simulink model "l64NM_actual"  (Simulink 7.9)
settings: Solver=ode45  StartTime=0.0  StopTime=1500000  RelTol=1e-3
undefined symbols (not defined in the file): pfb_coeff_gen_calc, sfp
libraries: xbsIndex_r4 x21822, casper_library_misc x651, casper_library_delays x192, xps_library x39, xbsReplacements_r4 x16, casper_library_pfbs x16, casper_library_ffts_twiddle x8, simulink x1
(part 1 of 315)
settings Model: Creator=jkocz  LastModifiedBy=jkocz
settings DebuggingCC: FcnCallInpInsideContextMsg=Use local settings  StrictBusMsg=Warning
settings GRTTargetCC: CombineOutputUpdateFcns=off  GRTInterface=on

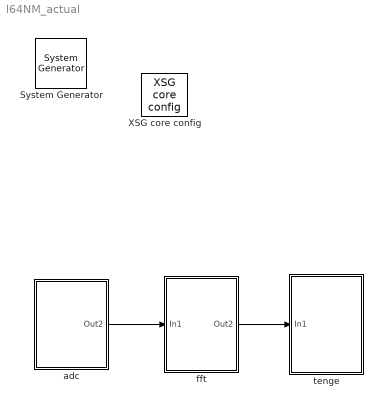
== system l64NM_actual ==
  " System Generator" : xbsIndex_r4/ System Generator  Tag=genX  xilinxfamily=virtex6  part=xc6vsx475t  speed=-1  package=ff1759  synthesis_tool=XST  clock_wrapper=Clock Enables  directory=./l64_actual/sysgen  proj_type=Project Navigator  Synth_file=XST Defaults  Impl_file=ISE Defaults  testbench=off  simulink_period=1  sysclk_period=5  dcm_input_clock_period=100  incr_netlist=off  trim_vbits=Everywhere in SubSystem  dbl_ovrd=According to Block Masks  core_generation=According to Block Masks  run_coregen=off  deprecated_control=off  eval_field=0  block_type=sysgen
      settings: block_version=11.4
  XSG core config : xps_library/XSG core config  Tag=xps:xsg  hw_sys=ROACH2:sx475t  clk_src=adc0_clk  ROACH_clk_src=adc0_clk  ROACH2_clk_src=adc0_clk  clk_rate=200  sample_period=1  synthesis_tool=XST
  adc : Subsystem
  fft : Subsystem
  tenge : Subsystem
  wires:
  adc/1(Out2) -> fft/1(In1)
  fft/1(Out2) -> tenge/1(In1)

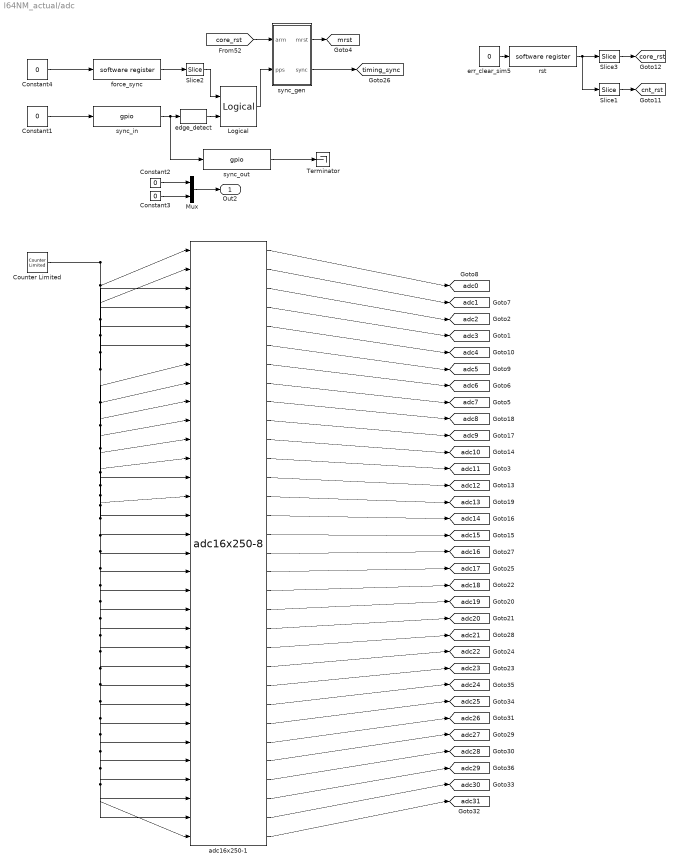
== system l64NM_actual/adc ==
  Constant1 : Constant  Value=0  SampleTime=1/4
  Constant2 : Constant  Value=0
  Constant3 : Constant  Value=0
  Constant4 : Constant  Value=0  SampleTime=1/4
  Counter Limited : simulink/Sources/Counter Limited  uplimit=4095  tsamp=-1
  Logical : xbsIndex_r4/Logical  logical_function=OR  inputs=2  en=off  latency=0  precision=Full  arith_type=Unsigned  n_bits=16  bin_pt=0  align_bp=on  dbl_ovrd=off  block_type=logical
  Mux : Mux  Inputs=2
  Slice1 : xbsIndex_r4/Slice  nbits=1  boolean_output=on  mode=Lower Bit Location + Width  bit1=0  base1=MSB of Input  bit0=0  base0=LSB of Input  dbl_ovrd=off  block_type=slice
      settings: block_version=10.1.3
  Slice2 : xbsIndex_r4/Slice  nbits=1  boolean_output=on  mode=Lower Bit Location + Width  bit1=0  base1=MSB of Input  bit0=0  base0=LSB of Input  dbl_ovrd=off  block_type=slice
      settings: block_version=10.1.3
  Slice3 : xbsIndex_r4/Slice  nbits=1  boolean_output=on  mode=Lower Bit Location + Width  bit1=0  base1=MSB of Input  bit0=1  base0=LSB of Input  dbl_ovrd=off  block_type=slice
      settings: block_version=10.1.3
  Terminator : Terminator
  adc16x250-1 : xps_library/ADCs/adc16x250-8  Tag=xps:adc16  board_count=2  roach2_rev=2
      settings: AttributesFormatString=r2 rev%<roach2_rev>
  edge_detect : casper_library_misc/edge_detect  edge=Rising  polarity=Active High  x_in=[0 0.08 0.08 0.16 0.16 0.24 0.24 0.32 0.32 0.4]  y_in="[-1 -1  1  1  1  1  1  1  1  1]"  x_out=[0.6 0.68 0.68 0.76 0.76 0.84 0.84 0.92 0.92 1]  y_out="[-1 -1  1  1 -1 -1 -1 -1 -1 -1]"
  err_clear_sim5 : Constant  Value=0
  force_sync : xps_library/software register  Tag=xps:sw_reg  io_dir=From Processor  arith_type=Unsigned  bitwidth=32  bin_pt=0  sample_period=1  gw_name=l64_actual_adc_force_sync_user_data_out
      link override r_10gbe_demo_gbe_core_rst_user_data_out: arith_type=Unsigned  n_bits=32  bin_pt=0  period=1
      link override r_10gbe_test_cnt_rst_user_data_out: arith_type=Unsigned  n_bits=32  bin_pt=0  period=1
      link override r_10gbe_test_gbe_core_rst_user_data_out: arith_type=Unsigned  n_bits=32  bin_pt=0  period=1
      link override r_xaui_test_cnt_rst_user_data_out: arith_type=Unsigned  n_bits=32  bin_pt=0  period=1
  rst : xps_library/software register  Tag=xps:sw_reg  io_dir=From Processor  arith_type=Unsigned  bitwidth=32  bin_pt=0  sample_period=1  gw_name=l64_actual_adc_rst_user_data_out
      link override r_10gbe_demo_gbe_core_rst_user_data_out: arith_type=Unsigned  n_bits=32  bin_pt=0  period=1
      link override r_10gbe_test_cnt_rst_user_data_out: arith_type=Unsigned  n_bits=32  bin_pt=0  period=1
      link override r_10gbe_test_gbe_core_rst_user_data_out: arith_type=Unsigned  n_bits=32  bin_pt=0  period=1
      link override r_xaui_test_cnt_rst_user_data_out: arith_type=Unsigned  n_bits=32  bin_pt=0  period=1
  sync_gen : Subsystem  mask(sync_gen_hw){sync_period_bits=27}
      mask prompts: sync_period_bits "Size of sync generator counter (bits)"
      mask help:
      | This circuit implements a scheme for synchronizing a design to within an FPGA clock of an external 1 pulse-per-second signal.
      |
      | * An arm pulse will cause the sync_gen to stop issuing sync pulses.
      | * It will then ignore the next input trigger (1PPS), while sync is flushed through the DSP pipeline.
      | * It will then lock onto the next trigger (1PPS) and issue syncs thereafter every sync_period clocks.
      | * You will need a multiple of (pfb_fir taps*LCM(FFT reorder orders) *fft bins/parallel inputs). See the sync memo for further details.
  sync_in : xps_library/gpio  Tag=xps:gpio  io_group=ROACH2:sync_in  io_dir=in  arith_type=Boolean  bitwidth=1  bin_pt=0  bit_index=0  sample_period=1  use_single_ended=off  use_ddr=off  reg_iob=on  reg_clk_phase=0  termination=None
  sync_out : xps_library/gpio  Tag=xps:gpio  io_group=ROACH2:sync_out  io_dir=out  arith_type=Boolean  bitwidth=1  bin_pt=0  bit_index=0  sample_period=1  use_single_ended=off  use_ddr=off  reg_iob=on  reg_clk_phase=0  termination=None
  Out2 : Outport
  tag scope: adc0=global, adc10=global, adc11=global, adc12=global, adc13=global, adc14=global, adc15=global, adc16=global, adc17=global, adc18=global, adc19=global, adc1=global, adc20=global, adc21=global, adc22=global, adc23=global, adc24=global, adc25=global, adc26=global, adc27=global, adc28=global, adc29=global, adc2=global, adc30=global, adc31=global, adc3=global, adc4=global, adc5=global, adc6=global, adc7=global, adc8=global, adc9=global, cnt_rst=global, core_rst=global, mrst=global, timing_sync=global
  wires:
  sync_gen/1(mrst) -> [mrst]
  sync_gen/2(sync) -> [timing_sync]
  [core_rst] -> sync_gen/1(arm)
  Mux/1 -> Out2/1
  Constant2/1 -> Mux/1
  Constant3/1 -> Mux/2
  err_clear_sim5/1 -> rst/1
  rst/1 -> Slice3/1, Slice1/1
  Slice1/1 -> [cnt_rst]
  Slice3/1 -> [core_rst]
  force_sync/1 -> Slice2/1
  Constant4/1 -> force_sync/1
  adc16x250-1/1 -> [adc0]
  Counter Limited/1 -> adc16x250-1/31, adc16x250-1/32, adc16x250-1/30, adc16x250-1/29, adc16x250-1/28, adc16x250-1/27, adc16x250-1/26, adc16x250-1/25, adc16x250-1/24, adc16x250-1/23, adc16x250-1/22, adc16x250-1/21, adc16x250-1/20, adc16x250-1/19, adc16x250-1/18, adc16x250-1/17, adc16x250-1/16, adc16x250-1/15, adc16x250-1/14, adc16x250-1/13, adc16x250-1/12, adc16x250-1/11, adc16x250-1/10, adc16x250-1/9, adc16x250-1/8, adc16x250-1/7, adc16x250-1/6, adc16x250-1/5, adc16x250-1/4, adc16x250-1/3, adc16x250-1/2, adc16x250-1/1
  adc16x250-1/2 -> [adc1]
  adc16x250-1/3 -> [adc2]
  adc16x250-1/4 -> [adc3]
  adc16x250-1/5 -> [adc4]
  adc16x250-1/6 -> [adc5]
  adc16x250-1/7 -> [adc6]
  adc16x250-1/8 -> [adc7]
  adc16x250-1/9 -> [adc8]
  adc16x250-1/10 -> [adc9]
  adc16x250-1/11 -> [adc10]
  adc16x250-1/12 -> [adc11]
  adc16x250-1/13 -> [adc12]
  adc16x250-1/14 -> [adc13]
  adc16x250-1/15 -> [adc14]
  adc16x250-1/16 -> [adc15]
  adc16x250-1/17 -> [adc16]
  adc16x250-1/18 -> [adc17]
  adc16x250-1/19 -> [adc18]
  adc16x250-1/20 -> [adc19]
  adc16x250-1/21 -> [adc20]
  adc16x250-1/22 -> [adc21]
  adc16x250-1/23 -> [adc22]
  adc16x250-1/24 -> [adc23]
  adc16x250-1/25 -> [adc24]
  adc16x250-1/26 -> [adc25]
  adc16x250-1/27 -> [adc26]
  adc16x250-1/28 -> [adc27]
  adc16x250-1/29 -> [adc28]
  adc16x250-1/30 -> [adc29]
  adc16x250-1/31 -> [adc30]
  adc16x250-1/32 -> [adc31]
  Constant1/1 -> sync_in/1
  Logical/1 -> sync_gen/2(pps)
  sync_in/1 -> sync_out/1, edge_detect/1
  sync_out/1 -> Terminator/1
  edge_detect/1 -> Logical/2
  Slice2/1 -> Logical/1

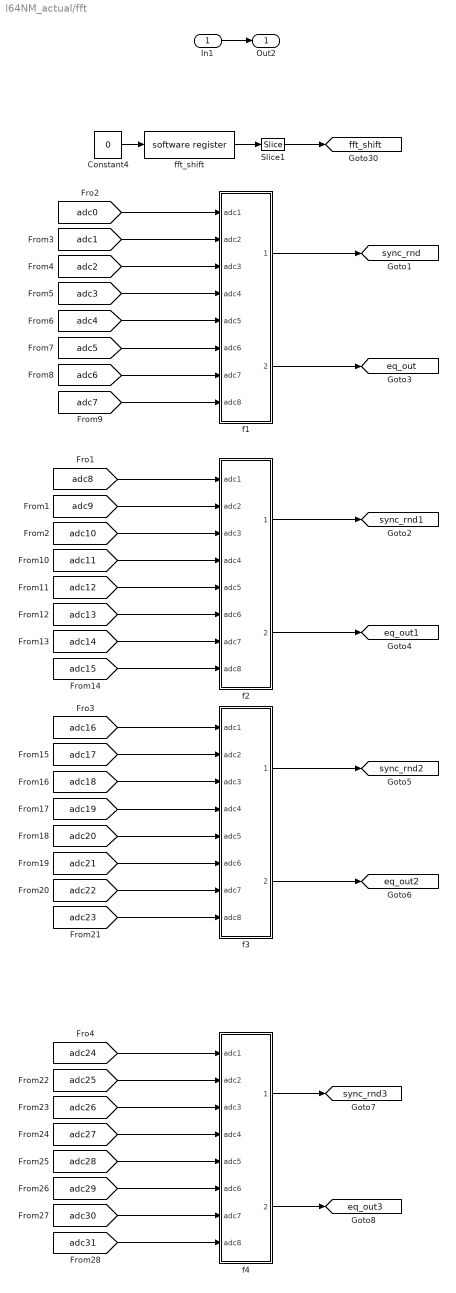
== system l64NM_actual/fft ==
  In1 : Inport
  Constant4 : Constant  Value=0  SampleTime=1/4
  Slice1 : xbsIndex_r4/Slice  nbits=16  boolean_output=off  mode=Lower Bit Location + Width  bit1=0  base1=MSB of Input  bit0=0  base0=LSB of Input  dbl_ovrd=off  block_type=slice
      settings: block_version=10.1.3
  f1 : Subsystem
  f2 : Subsystem
  f3 : Subsystem
  f4 : Subsystem
  fft_shift : xps_library/software register  Tag=xps:sw_reg  io_dir=From Processor  arith_type=Unsigned  bitwidth=32  bin_pt=0  sample_period=1  gw_name=l64_actual_fft_fft_shift_user_data_out
      link override r_10gbe_demo_gbe_core_rst_user_data_out: arith_type=Unsigned  n_bits=32  bin_pt=0  period=1
      link override r_10gbe_test_cnt_rst_user_data_out: arith_type=Unsigned  n_bits=32  bin_pt=0  period=1
      link override r_10gbe_test_gbe_core_rst_user_data_out: arith_type=Unsigned  n_bits=32  bin_pt=0  period=1
      link override r_xaui_test_cnt_rst_user_data_out: arith_type=Unsigned  n_bits=32  bin_pt=0  period=1
  Out2 : Outport
  tag scope: eq_out1=global, eq_out2=global, eq_out3=global, eq_out=global, fft_shift=global, sync_rnd1=global, sync_rnd2=global, sync_rnd3=global, sync_rnd=global
  wires:
  In1/1 -> Out2/1
  fft_shift/1 -> Slice1/1
  Constant4/1 -> fft_shift/1
  Slice1/1 -> [fft_shift]
  [adc0] -> f1/1(adc1)
  [adc1] -> f1/2(adc2)
  f1/1(sync_rnd) -> [sync_rnd]
  f1/2(eq_out) -> [eq_out]
  [adc2] -> f1/3(adc3)
  [adc3] -> f1/4(adc4)
  [adc4] -> f1/5(adc5)
  [adc5] -> f1/6(adc6)
  [adc6] -> f1/7(adc7)
  [adc7] -> f1/8(adc8)
  f2/1(sync_rnd) -> [sync_rnd1]
  f2/2(eq_out) -> [eq_out1]
  [adc8] -> f2/1(adc1)
  [adc9] -> f2/2(adc2)
  [adc10] -> f2/3(adc3)
  [adc11] -> f2/4(adc4)
  [adc12] -> f2/5(adc5)
  [adc13] -> f2/6(adc6)
  [adc14] -> f2/7(adc7)
  [adc15] -> f2/8(adc8)
  [adc16] -> f3/1(adc1)
  [adc17] -> f3/2(adc2)
  [adc18] -> f3/3(adc3)
  [adc19] -> f3/4(adc4)
  [adc20] -> f3/5(adc5)
  [adc21] -> f3/6(adc6)
  [adc22] -> f3/7(adc7)
  [adc23] -> f3/8(adc8)
  f3/1(sync_rnd) -> [sync_rnd2]
  f3/2(eq_out) -> [eq_out2]
  f4/1(sync_rnd) -> [sync_rnd3]
  f4/2(eq_out) -> [eq_out3]
  [adc24] -> f4/1(adc1)
  [adc25] -> f4/2(adc2)
  [adc26] -> f4/3(adc3)
  [adc27] -> f4/4(adc4)
  [adc28] -> f4/5(adc5)
  [adc29] -> f4/6(adc6)
  [adc30] -> f4/7(adc7)
  [adc31] -> f4/8(adc8)

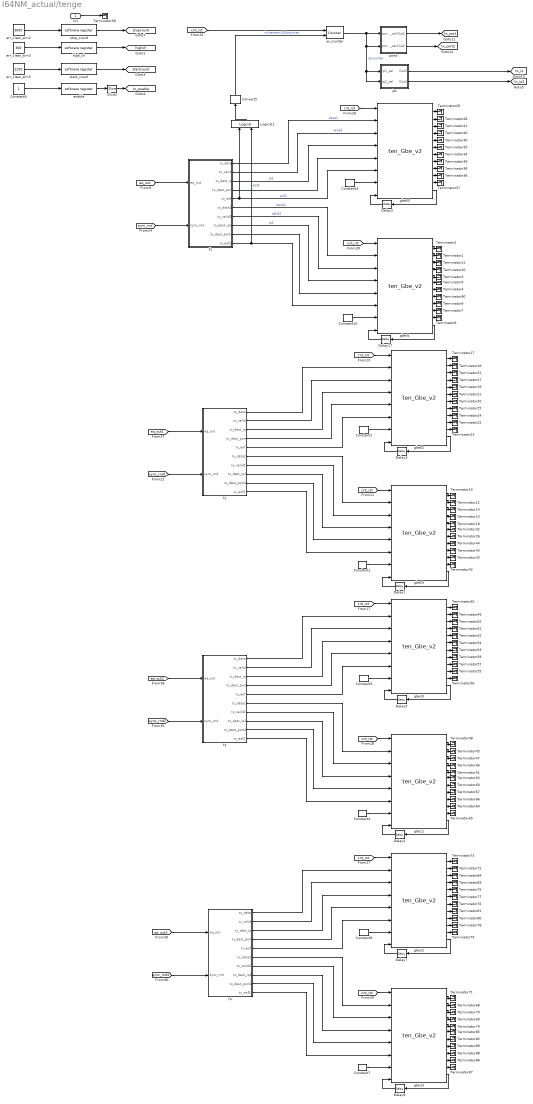
== system l64NM_actual/tenge ==
  In1 : Inport
  Constant{1..5,7..8,10} (8 alike) : xbsIndex_r4/Constant  const=1  gui_display_data_type=Fixed-point  arith_type=Boolean  n_bits=1  bin_pt=0  preci_type=Single  exp_width=8  frac_width=24  explicit_period=on  period=1  equ=P=C  opselect=C  inp2=PCIN>>17  opr=+  inp1=P  carry=CIN  dbl_ovrd=off  block_type=constant
  Constant6 : Constant
  Convert5 : xbsIndex_r4/Convert  gui_display_data_type=Boolean  arith_type=Boolean  n_bits=64  bin_pt=0  float_type=Single  exp_bits=8  fraction_bits=24  quantization=Truncate  overflow=Wrap  en=off  latency=0  dbl_ovrd=off  pipeline=off  block_type=convert
      settings: block_version=11.4
  Delay{1..7,17} (8 alike) : xbsIndex_r4/Delay  rst=off  infoeditControl=Selection of Reset will increase slice count due to use of real FFs and instead of SRLs  en=off  latency=1  dbl_ovrd=off  reg_retiming=on  block_type=delay
  Logical1 : xbsIndex_r4/Logical  logical_function=OR  inputs=2  en=off  latency=1  precision=Full  arith_type=Unsigned  n_bits=16  bin_pt=0  align_bp=on  dbl_ovrd=off  block_type=logical
      settings: block_version=11.4
  Slice2 : xbsIndex_r4/Slice  nbits=1  boolean_output=off  mode=Lower Bit Location + Width  bit1=1  base1=MSB of Input  bit0=0  base0=LSB of Input  dbl_ovrd=off  block_type=slice
      settings: block_version=10.1.3
  Terminator{1..5,7..90} (89 alike) : Terminator
  enable : xps_library/software register  Tag=xps:sw_reg  io_dir=From Processor  arith_type=Unsigned  bitwidth=32  bin_pt=0  sample_period=1  gw_name=l64_actual_tenge_enable_user_data_out
      link override r_10gbe_demo_pkt_sim_run_user_data_out: arith_type=Unsigned  n_bits=32  bin_pt=0  period=1
      link override r_10gbe_test_pkt_sim_run_user_data_out: arith_type=Unsigned  n_bits=32  bin_pt=0  period=1
      link override test_pkt_buffer_10_1_p_mux_up_run_user_data_out: arith_type=Unsigned  n_bits=32  bin_pt=0  period=1
  err_clear_sim2 : Constant  Value=3650
  err_clear_sim3 : Constant  Value=300
  err_clear_sim5 : Constant  Value=1250
  f1 : Subsystem
  f2 : Subsystem
  f3 : Subsystem
  f4 : Subsystem
  gbe00 : xps_library/ten_Gbe_v2  Tag=xps:tengbe_v2  flavour=sfp+  slot=0  port_r1=0  port_r2_cx4=0  port_r2_sfpp=0  rx_dist_ram=off  large_frames=on  show_param=on  pre_emph_r2=0.74  pre_emph=3  post_emph_r2=0.18  rxeqmix_r2=7  swing_r2=940  swing=800  fab_en=off  fab_mac=hex2dec('123456789100')  fab_ip=192*(2^24) + 168*(2^16) + 5*(2^8) + 14*(2^0)  fab_udp=10002  fab_gate=192*(2^24) + 168*(2^16) + 5*(2^8) + 0  cpu_rx_en=on  cpu_tx_en=on
  gbe01 : xps_library/ten_Gbe_v2  Tag=xps:tengbe_v2  flavour=sfp+  slot=0  port_r1=0  port_r2_cx4=0  port_r2_sfpp=1  rx_dist_ram=off  large_frames=on  show_param=on  pre_emph_r2=0.74  pre_emph=3  post_emph_r2=0.18  rxeqmix_r2=7  swing_r2=940  swing=800  fab_en=off  fab_mac=hex2dec('123456789105')  fab_ip=192*(2^24) + 168*(2^16) + 6*(2^8) + 15*(2^0)  fab_udp=10002  fab_gate=192*(2^24) + 168*(2^16) + 6*(2^8) + 0  cpu_rx_en=on  cpu_tx_en=on
  gbe02 : xps_library/ten_Gbe_v2  Tag=xps:tengbe_v2  flavour=sfp+  slot=0  port_r1=0  port_r2_cx4=0  port_r2_sfpp=2  rx_dist_ram=off  large_frames=on  show_param=on  pre_emph_r2=0.74  pre_emph=3  post_emph_r2=0.18  rxeqmix_r2=7  swing_r2=940  swing=800  fab_en=off  fab_mac=hex2dec('123456789100')  fab_ip=192*(2^24) + 168*(2^16) + 5*(2^8) + 14*(2^0)  fab_udp=10002  fab_gate=192*(2^24) + 168*(2^16) + 5*(2^8) + 0  cpu_rx_en=on  cpu_tx_en=on
  gbe03 : xps_library/ten_Gbe_v2  Tag=xps:tengbe_v2  flavour=sfp+  slot=0  port_r1=0  port_r2_cx4=0  port_r2_sfpp=3  rx_dist_ram=off  large_frames=on  show_param=on  pre_emph_r2=0.74  pre_emph=3  post_emph_r2=0.18  rxeqmix_r2=7  swing_r2=940  swing=800  fab_en=off  fab_mac=hex2dec('123456789105')  fab_ip=192*(2^24) + 168*(2^16) + 6*(2^8) + 15*(2^0)  fab_udp=10002  fab_gate=192*(2^24) + 168*(2^16) + 6*(2^8) + 0  cpu_rx_en=on  cpu_tx_en=on
  gbe10 : xps_library/ten_Gbe_v2  Tag=xps:tengbe_v2  flavour=sfp+  slot=1  port_r1=0  port_r2_cx4=0  port_r2_sfpp=0  rx_dist_ram=off  large_frames=on  show_param=on  pre_emph_r2=0.74  pre_emph=3  post_emph_r2=0.18  rxeqmix_r2=7  swing_r2=940  swing=800  fab_en=off  fab_mac=hex2dec('123456789100')  fab_ip=192*(2^24) + 168*(2^16) + 5*(2^8) + 14*(2^0)  fab_udp=10002  fab_gate=192*(2^24) + 168*(2^16) + 5*(2^8) + 0  cpu_rx_en=on  cpu_tx_en=on
  gbe11 : xps_library/ten_Gbe_v2  Tag=xps:tengbe_v2  flavour=sfp+  slot=1  port_r1=0  port_r2_cx4=0  port_r2_sfpp=1  rx_dist_ram=off  large_frames=on  show_param=on  pre_emph_r2=0.74  pre_emph=3  post_emph_r2=0.18  rxeqmix_r2=7  swing_r2=940  swing=800  fab_en=off  fab_mac=hex2dec('123456789105')  fab_ip=192*(2^24) + 168*(2^16) + 6*(2^8) + 15*(2^0)  fab_udp=10002  fab_gate=192*(2^24) + 168*(2^16) + 6*(2^8) + 0  cpu_rx_en=on  cpu_tx_en=on
  gbe12 : xps_library/ten_Gbe_v2  Tag=xps:tengbe_v2  flavour=sfp+  slot=1  port_r1=0  port_r2_cx4=0  port_r2_sfpp=2  rx_dist_ram=off  large_frames=on  show_param=on  pre_emph_r2=0.74  pre_emph=3  post_emph_r2=0.18  rxeqmix_r2=7  swing_r2=940  swing=800  fab_en=off  fab_mac=hex2dec('123456789100')  fab_ip=192*(2^24) + 168*(2^16) + 5*(2^8) + 14*(2^0)  fab_udp=10002  fab_gate=192*(2^24) + 168*(2^16) + 5*(2^8) + 0  cpu_rx_en=on  cpu_tx_en=on
  gbe13 : xps_library/ten_Gbe_v2  Tag=xps:tengbe_v2  flavour=sfp+  slot=1  port_r1=0  port_r2_cx4=0  port_r2_sfpp=3  rx_dist_ram=off  large_frames=on  show_param=on  pre_emph_r2=0.74  pre_emph=3  post_emph_r2=0.18  rxeqmix_r2=7  swing_r2=940  swing=800  fab_en=off  fab_mac=hex2dec('123456789105')  fab_ip=192*(2^24) + 168*(2^16) + 6*(2^8) + 15*(2^0)  fab_udp=10002  fab_gate=192*(2^24) + 168*(2^16) + 6*(2^8) + 0  cpu_rx_en=on  cpu_tx_en=on
  high_ch : xps_library/software register  Tag=xps:sw_reg  io_dir=From Processor  arith_type=Unsigned  bitwidth=32  bin_pt=0  sample_period=1  gw_name=l64_actual_tenge_high_ch_user_data_out
      link override r_10gbe_demo_dest_ip_user_data_out: arith_type=Unsigned  n_bits=32  bin_pt=0  period=1
      link override r_10gbe_test_dest_ip_user_data_out: arith_type=Unsigned  n_bits=32  bin_pt=0  period=1
      link override r_xaui_test_cnt_rst_user_data_out: arith_type=Unsigned  n_bits=32  bin_pt=0  period=1
  ip_counter : xbsIndex_r4/Counter  cnt_type=Free Running  cnt_to=Inf  operation=Up  start_count=0  cnt_by_val=1  arith_type=Unsigned  n_bits=3  bin_pt=0  load_pin=off  rst=on  en=on  explicit_period=on  period=1  dbl_ovrd=off  use_behavioral_HDL=off  implementation=Fabric  block_type=counter
      settings: block_version=11.4
  ips : Subsystem
  ports : Subsystem
  start_count : xps_library/software register  Tag=xps:sw_reg  io_dir=From Processor  arith_type=Unsigned  bitwidth=32  bin_pt=0  sample_period=1  gw_name=l64_actual_tenge_start_count_user_data_out
      link override r_10gbe_demo_dest_ip_user_data_out: arith_type=Unsigned  n_bits=32  bin_pt=0  period=1
      link override r_10gbe_test_dest_ip_user_data_out: arith_type=Unsigned  n_bits=32  bin_pt=0  period=1
      link override r_xaui_test_cnt_rst_user_data_out: arith_type=Unsigned  n_bits=32  bin_pt=0  period=1
  stop_count : xps_library/software register  Tag=xps:sw_reg  io_dir=From Processor  arith_type=Unsigned  bitwidth=32  bin_pt=0  sample_period=1  gw_name=l64_actual_tenge_stop_count_user_data_out
      link override leda_64_sim_tenge_stop_count_user_data_out: arith_type=Unsigned  n_bits=32  bin_pt=0  period=1
      link override r_10gbe_demo_dest_ip_user_data_out: arith_type=Unsigned  n_bits=32  bin_pt=0  period=1
      link override r_10gbe_test_dest_ip_user_data_out: arith_type=Unsigned  n_bits=32  bin_pt=0  period=1
      link override r_xaui_test_cnt_rst_user_data_out: arith_type=Unsigned  n_bits=32  bin_pt=0  period=1
  tag scope: highch=global, startcount=global, stopcount=global, tx_enable=global, tx_ip2=global, tx_ip=global, tx_port2=global, tx_port=global
  wires:
  In1/1 -> Terminator58/1
  gbe02/12 -> Delay3/1
  Delay3/1 -> gbe02/8
  gbe02/5 -> Terminator19/1
  gbe02/4 -> Terminator27/1
  gbe02/9 -> Terminator24/1
  gbe02/1 -> Terminator17/1
  gbe02/2 -> Terminator16/1
  gbe02/3 -> Terminator31/1
  gbe02/6 -> Terminator21/1
  gbe02/7 -> Terminator20/1
  gbe02/8 -> Terminator25/1
  gbe02/10 -> Terminator22/1
  gbe02/11 -> Terminator23/1
  [cnt_rst] -> gbe02/1
  Constant1/1 -> gbe03/7
  gbe03/12 -> Delay1/1
  Delay1/1 -> gbe03/8
  gbe03/5 -> Terminator18/1
  gbe03/4 -> Terminator13/1
  gbe03/9 -> Terminator43/1
  gbe03/1 -> Terminator15/1
  gbe03/2 -> Terminator12/1
  gbe03/3 -> Terminator14/1
  gbe03/6 -> Terminator32/1
  gbe03/7 -> Terminator26/1
  gbe03/8 -> Terminator44/1
  gbe03/10 -> Terminator33/1
  gbe03/11 -> Terminator42/1
  [cnt_rst] -> gbe03/1
  Constant2/1 -> gbe02/7
  f2/1(tx_data) -> gbe02/2
  f2/2(tx_valid) -> gbe02/3
  f2/3(tx_dest_ip) -> gbe02/4
  f2/4(tx_dest_port) -> gbe02/5
  f2/5(tx_eof) -> gbe02/6
  f2/6(tx_data2) -> gbe03/2
  f2/7(tx_valid2) -> gbe03/3
  f2/8(tx_dest_ip2) -> gbe03/4
  f2/9(tx_dest_port2) -> gbe03/5
  f2/10(tx_eof2) -> gbe03/6
  gbe10/12 -> Delay5/1
  Delay5/1 -> gbe10/8
  gbe10/5 -> Terminator52/1
  gbe10/4 -> Terminator61/1
  gbe10/9 -> Terminator57/1
  gbe10/1 -> Terminator50/1
  gbe10/2 -> Terminator49/1
  gbe10/3 -> Terminator62/1
  gbe10/6 -> Terminator54/1
  gbe10/7 -> Terminator53/1
  gbe10/8 -> Terminator59/1
  gbe10/10 -> Terminator55/1
  gbe10/11 -> Terminator56/1
  [cnt_rst] -> gbe10/1
  Constant4/1 -> gbe11/7
  gbe11/12 -> Delay4/1
  Delay4/1 -> gbe11/8
  gbe11/5 -> Terminator51/1
  gbe11/4 -> Terminator46/1
  gbe11/9 -> Terminator66/1
  gbe11/1 -> Terminator48/1
  gbe11/2 -> Terminator45/1
  gbe11/3 -> Terminator47/1
  gbe11/6 -> Terminator63/1
  gbe11/7 -> Terminator60/1
  gbe11/8 -> Terminator67/1
  gbe11/10 -> Terminator64/1
  gbe11/11 -> Terminator65/1
  [cnt_rst] -> gbe11/1
  Constant5/1 -> gbe10/7
  f3/1(tx_data) -> gbe10/2
  f3/2(tx_valid) -> gbe10/3
  f3/3(tx_dest_ip) -> gbe10/4
  f3/4(tx_dest_port) -> gbe10/5
  f3/5(tx_eof) -> gbe10/6
  f3/6(tx_data2) -> gbe11/2
  f3/7(tx_valid2) -> gbe11/3
  f3/8(tx_dest_ip2) -> gbe11/4
  f3/9(tx_dest_port2) -> gbe11/5
  f3/10(tx_eof2) -> gbe11/6
  gbe12/12 -> Delay7/1
  Delay7/1 -> gbe12/8
  gbe12/5 -> Terminator75/1
  gbe12/4 -> Terminator83/1
  gbe12/9 -> Terminator80/1
  gbe12/1 -> Terminator73/1
  gbe12/2 -> Terminator72/1
  gbe12/3 -> Terminator84/1
  gbe12/6 -> Terminator77/1
  gbe12/7 -> Terminator76/1
  gbe12/8 -> Terminator81/1
  gbe12/10 -> Terminator78/1
  gbe12/11 -> Terminator79/1
  [cnt_rst] -> gbe12/1
  Constant7/1 -> gbe13/7
  gbe13/12 -> Delay6/1
  Delay6/1 -> gbe13/8
  gbe13/5 -> Terminator74/1
  gbe13/4 -> Terminator69/1
  gbe13/9 -> Terminator88/1
  gbe13/1 -> Terminator71/1
  gbe13/2 -> Terminator68/1
  gbe13/3 -> Terminator70/1
  gbe13/6 -> Terminator85/1
  gbe13/7 -> Terminator82/1
  gbe13/8 -> Terminator89/1
  gbe13/10 -> Terminator86/1
  gbe13/11 -> Terminator87/1
  [cnt_rst] -> gbe13/1
  Constant8/1 -> gbe12/7
  f4/1(tx_data) -> gbe12/2
  f4/2(tx_valid) -> gbe12/3
  f4/3(tx_dest_ip) -> gbe12/4
  f4/4(tx_dest_port) -> gbe12/5
  f4/5(tx_eof) -> gbe12/6
  f4/6(tx_data2) -> gbe13/2
  f4/7(tx_valid2) -> gbe13/3
  f4/8(tx_dest_ip2) -> gbe13/4
  f4/9(tx_dest_port2) -> gbe13/5
  f4/10(tx_eof2) -> gbe13/6
  [eq_out] -> f1/1(eq_out)
  err_clear_sim2/1 -> stop_count/1
  stop_count/1 -> [stopcount]
  err_clear_sim3/1 -> high_ch/1
  high_ch/1 -> [highch]
  err_clear_sim5/1 -> start_count/1
  start_count/1 -> [startcount]
  enable/1 -> Slice2/1
  Constant6/1 -> enable/1
  Slice2/1 -> [tx_enable]
  [sync_rnd] -> f1/2(sync_rnd)
  gbe00/12 -> Delay2/1
  Delay2/1 -> gbe00/8
  gbe00/5 -> Terminator30/1
  gbe00/4 -> Terminator40/1
  gbe00/9 -> Terminator38/1
  gbe00/1 -> Terminator29/1
  gbe00/2 -> Terminator28/1
  gbe00/3 -> Terminator41/1
  gbe00/6 -> Terminator35/1
  gbe00/7 -> Terminator34/1
  gbe00/8 -> Terminator39/1
  gbe00/10 -> Terminator36/1
  gbe00/11 -> Terminator37/1
  [cnt_rst] -> gbe00/1
  Constant10/1 -> gbe01/7
  gbe01/12 -> Delay17/1
  Delay17/1 -> gbe01/8
  gbe01/5 -> Terminator3/1
  gbe01/4 -> Terminator10/1
  gbe01/9 -> Terminator9/1
  gbe01/1 -> Terminator2/1
  gbe01/2 -> Terminator1/1
  gbe01/3 -> Terminator11/1
  gbe01/6 -> Terminator5/1
  gbe01/7 -> Terminator4/1
  gbe01/8 -> Terminator90/1
  gbe01/10 -> Terminator7/1
  gbe01/11 -> Terminator8/1
  [cnt_rst] -> gbe01/1
  Constant3/1 -> gbe00/7
  f1/1(tx_data) -> gbe00/2  "data1"
  f1/2(tx_valid) -> gbe00/3  "valid1"
  f1/3(tx_dest_ip) -> gbe00/4  "ip1"
  f1/4(tx_dest_port) -> gbe00/5
  f1/5(tx_eof) -> Logical1/1, gbe00/6  "eof1"
  f1/6(tx_data2) -> gbe01/2  "data2"
  f1/7(tx_valid2) -> gbe01/3  "valid2"
  f1/8(tx_dest_ip2) -> gbe01/4  "ip2"
  f1/9(tx_dest_port2) -> gbe01/5
  f1/10(tx_eof2) -> Logical1/2, gbe01/6  "eof2"
  ports/1(Out1) -> [tx_port]
  ports/2(Out2) -> [tx_port2]
  ips/1(Out1) -> [tx_ip]
  ips/2(Out2) -> [tx_ip2]
  ip_counter/1 -> ports/1(port1_sel), ports/2(port2_sel), ips/1(ip1_sel), ips/2(ip2_sel)  "ipcounter"
  [cnt_rst] -> ip_counter/1
  Logical1/1 -> Convert5/1
  Convert5/1 -> ip_counter/2  "increment300counter"
  [eq_out1] -> f2/1(eq_out)
  [sync_rnd1] -> f2/2(sync_rnd)
  [eq_out2] -> f3/1(eq_out)
  [sync_rnd2] -> f3/2(sync_rnd)
  [eq_out3] -> f4/1(eq_out)
  [sync_rnd3] -> f4/2(sync_rnd)

== system l64NM_actual/adc/sync_gen ==
  arm : Inport
  pps : Inport  Port=2
  Constant : xbsIndex_r4/Constant  const=2^sync_period_bits  gui_display_data_type=Fixed-point  arith_type=Unsigned  n_bits=sync_period_bits+1  bin_pt=0  preci_type=Single  exp_width=8  frac_width=24  explicit_period=on  period=1  equ=P=C  opselect=C  inp2=PCIN>>17  opr=+  inp1=P  carry=CIN  dbl_ovrd=off  block_type=constant
  Delay1 : xbsIndex_r4/Delay  rst=off  infoeditControl=Selection of Reset will increase slice count due to use of real FFs and instead of SRLs  en=off  latency=1  dbl_ovrd=off  reg_retiming=on  block_type=delay
  Delay2 : xbsIndex_r4/Delay  rst=off  infoeditControl=Selection of Reset will increase slice count due to use of real FFs and instead of SRLs  en=off  latency=1  dbl_ovrd=off  reg_retiming=on  block_type=delay
  rst_gen : Subsystem  mask{reset_period=1}
      mask prompts: reset_period "Min period of reset (pps pulses)"
      mask init:
      | %reset_period = eval(get_param(gcb,'reset_period'))
      | %bits = ceil(log2(reset_period))
      | %set_param([gcb,'/Counter'],'n_bits',num2str(bits));
      | %set_param([gcb,'/Counter'],'start_count', num2str(reset_period))
      | %set_param([gcb,'/Constant'],'n_bits',num2str(bits));
  sync_gen : Subsystem  mask{sync_gen_bits=sync_period_bits+1}
      mask prompts: sync_gen_bits "Size of sync generator counter"
  mrst : Outport
  sync : Outport  Port=2
  wires:
  arm/1 -> rst_gen/1(arm)
  pps/1 -> rst_gen/2(sync_in)
  sync_gen/1(sync) -> Delay2/1
  rst_gen/1(mrst) -> sync_gen/1(rst), Delay1/1
  Delay1/1 -> mrst/1
  Delay2/1 -> sync/1
  Constant/1 -> sync_gen/2(sync_period)
